simulink model "isi_correlator"  (Simulink 7.0)
settings: Solver=ode45  StartTime=0.0  StopTime=2500  RelTol=1e-3
undefined symbols (not defined in the file): bit_rev, pfb_coeff_gen_calc
libraries: xbsIndex_r4 x4490, casper_library x399, xbsReplacements_r4 x27, isi_correlator_lib x8, xps_library x7, dspsigops x2, simulink x1
(part 2 of 49)

== system isi_correlator/cram2 ==
  In1 : Inport  OutDataType=sfix(16)  OutScaling=2^0
  In2 : Inport  Port=2  OutDataType=sfix(16)  OutScaling=2^0
  In3 : Inport  Port=3  OutDataType=sfix(16)  OutScaling=2^0
  In4 : Inport  Port=4
  In5 : Inport  Port=5
  In6 : Inport  Port=6
  In7 : Inport  Port=7
  In8 : Inport  Port=8
  In9 : Inport  Port=9
  Concat : xbsIndex_r4/Concat  num_inputs=9  dbl_ovrd=off  block_type=concat
      settings: block_version=10.1.2
  Reinterp{1..9} (9 alike) : xbsIndex_r4/Reinterpret  force_arith_type=on  arith_type=Unsigned  force_bin_pt=on  bin_pt=0  block_type=reinterpret
      Reinterp1 settings: infoedit="Changes signal type without altering the binary representation.   You can changed the signal between signed and unsigned, and relocate the binary point.<P><P>Hardware notes: In hardware this block costs nothing.<P><P>Example:  Suppose the input is 6 bits wide, signed, with 2 fractional bits, and the output is forced to unsigned with 0 fractional bits.  Then an input of -2.0 (1110.00 in binary 2's complement) becomes an output of 56 (111000 in binary)."  block_version=10.1.2
      Reinterp2 settings: infoedit="Changes signal type without altering the binary representation.   You can changed the signal between signed and unsigned, and relocate the binary point.<P><P>Hardware notes: In hardware this block costs nothing.<P><P>Example:  Suppose the input is 6 bits wide, signed, with 2 fractional bits, and the output is forced to unsigned with 0 fractional bits.  Then an input of -2.0 (1110.00 in binary 2's complement) becomes an output of 56 (111000 in binary)."  block_version=10.1.2
      Reinterp3 settings: infoedit="Changes signal type without altering the binary representation.   You can changed the signal between signed and unsigned, and relocate the binary point.<P><P>Hardware notes: In hardware this block costs nothing.<P><P>Example:  Suppose the input is 6 bits wide, signed, with 2 fractional bits, and the output is forced to unsigned with 0 fractional bits.  Then an input of -2.0 (1110.00 in binary 2's complement) becomes an output of 56 (111000 in binary)."  block_version=10.1.2
      Reinterp4 settings: infoedit="Changes signal type without altering the binary representation.   You can changed the signal between signed and unsigned, and relocate the binary point.<P><P>Hardware notes: In hardware this block costs nothing.<P><P>Example:  Suppose the input is 6 bits wide, signed, with 2 fractional bits, and the output is forced to unsigned with 0 fractional bits.  Then an input of -2.0 (1110.00 in binary 2's complement) becomes an output of 56 (111000 in binary)."  block_version=10.1.2
      Reinterp5 settings: infoedit="Changes signal type without altering the binary representation.   You can changed the signal between signed and unsigned, and relocate the binary point.<P><P>Hardware notes: In hardware this block costs nothing.<P><P>Example:  Suppose the input is 6 bits wide, signed, with 2 fractional bits, and the output is forced to unsigned with 0 fractional bits.  Then an input of -2.0 (1110.00 in binary 2's complement) becomes an output of 56 (111000 in binary)."  block_version=10.1.3
      Reinterp6 settings: infoedit="Changes signal type without altering the binary representation.   You can changed the signal between signed and unsigned, and relocate the binary point.<P><P>Hardware notes: In hardware this block costs nothing.<P><P>Example:  Suppose the input is 6 bits wide, signed, with 2 fractional bits, and the output is forced to unsigned with 0 fractional bits.  Then an input of -2.0 (1110.00 in binary 2's complement) becomes an output of 56 (111000 in binary)."  block_version=10.1.3
      Reinterp7 settings: infoedit="Changes signal type without altering the binary representation.   You can changed the signal between signed and unsigned, and relocate the binary point.<P><P>Hardware notes: In hardware this block costs nothing.<P><P>Example:  Suppose the input is 6 bits wide, signed, with 2 fractional bits, and the output is forced to unsigned with 0 fractional bits.  Then an input of -2.0 (1110.00 in binary 2's complement) becomes an output of 56 (111000 in binary)."  block_version=10.1.3
      Reinterp8 settings: infoedit="Changes signal type without altering the binary representation.   You can changed the signal between signed and unsigned, and relocate the binary point.<P><P>Hardware notes: In hardware this block costs nothing.<P><P>Example:  Suppose the input is 6 bits wide, signed, with 2 fractional bits, and the output is forced to unsigned with 0 fractional bits.  Then an input of -2.0 (1110.00 in binary 2's complement) becomes an output of 56 (111000 in binary)."  block_version=10.1.3
      Reinterp9 settings: infoedit="Changes signal type without altering the binary representation.   You can changed the signal between signed and unsigned, and relocate the binary point.<P><P>Hardware notes: In hardware this block costs nothing.<P><P>Example:  Suppose the input is 6 bits wide, signed, with 2 fractional bits, and the output is forced to unsigned with 0 fractional bits.  Then an input of -2.0 (1110.00 in binary 2's complement) becomes an output of 56 (111000 in binary)."  block_version=10.1.3
  Out : Outport
  wires:
  Concat/1 -> Out/1
  In1/1 -> Reinterp1/1
  Reinterp1/1 -> Concat/1
  In2/1 -> Reinterp2/1
  Reinterp2/1 -> Concat/2
  In3/1 -> Reinterp3/1
  Reinterp3/1 -> Concat/3
  In4/1 -> Reinterp4/1
  Reinterp4/1 -> Concat/4
  In5/1 -> Reinterp5/1
  Reinterp5/1 -> Concat/5
  In6/1 -> Reinterp6/1
  Reinterp6/1 -> Concat/6
  In7/1 -> Reinterp7/1
  Reinterp7/1 -> Concat/7
  In8/1 -> Reinterp8/1
  Reinterp8/1 -> Concat/8
  In9/1 -> Reinterp9/1
  Reinterp9/1 -> Concat/9

== system isi_correlator/fft_wideband_real ==
  sync : Inport  OutDataType=sfix(16)  OutScaling=2^0
  shift : Inport  Port=2  OutDataType=sfix(16)  OutScaling=2^0
  in0 : Inport  Port=3  OutDataType=sfix(16)  OutScaling=2^0
  in1 : Inport  Port=4  OutDataType=sfix(16)  OutScaling=2^0
  in2 : Inport  Port=5  OutDataType=sfix(16)  OutScaling=2^0
  in3 : Inport  Port=6  OutDataType=sfix(16)  OutScaling=2^0
  in4 : Inport  Port=7
  in5 : Inport  Port=8
  in6 : Inport  Port=9
  in7 : Inport  Port=10
  in8 : Inport  Port=11
  in9 : Inport  Port=12
  in10 : Inport  Port=13
  in11 : Inport  Port=14
  in12 : Inport  Port=15
  in13 : Inport  Port=16
  in14 : Inport  Port=17
  in15 : Inport  Port=18
  fft_biplex_real_4x0 : Subsystem  AncestorBlock=casper_library/FFTs/fft_biplex_real_4x  mask(fft_biplex_real_4x){FFTSize=3, BitWidth=18, quantization=Truncate, overflow=Wrap, add_latency=2, mult_latency=2, bram_latency=3}
      mask prompts: FFTSize "Size of FFT: (2^? pnts)"; BitWidth "Bitwidth: (Max Efficiency at 18 bits)"; quantization "Quantization Behavior"; overflow "Overflow Behavior"; add_latency "Add Latency"; mult_latency "Mult Latency"; bram_latency "BRAM Latency"
      mask init:
      | fft_biplex_real_4x_init(gcb,...
      |     'FFTSize', FFTSize,...
      |     'BitWidth', BitWidth,...
      |     'quantization', quantization,...
      |     'overflow', overflow,...
      |     'add_latency', add_latency,...
      |     'mult_latency', mult_latency,...
      |     'bram_latency', bram_latency);
      settings: AttributesFormatString=FFTSize=3, BitWidth=18
      mask StyleString: edit,edit,popup(Truncate|Round  (unbiased: +/- Inf)|Round  (unbiased: Even Values)),popup(Wrap|Saturate|Error),edit,edit,edit
  fft_biplex_real_4x1 : Subsystem  AncestorBlock=casper_library/FFTs/fft_biplex_real_4x  mask(fft_biplex_real_4x){FFTSize=3, BitWidth=18, quantization=Truncate, overflow=Wrap, add_latency=2, mult_latency=2, bram_latency=3}
      mask prompts: FFTSize "Size of FFT: (2^? pnts)"; BitWidth "Bitwidth: (Max Efficiency at 18 bits)"; quantization "Quantization Behavior"; overflow "Overflow Behavior"; add_latency "Add Latency"; mult_latency "Mult Latency"; bram_latency "BRAM Latency"
      mask init: same as isi_correlator/fft_wideband_real/fft_biplex_real_4x0
      settings: AttributesFormatString=FFTSize=3, BitWidth=18
      mask StyleString: edit,edit,popup(Truncate|Round  (unbiased: +/- Inf)|Round  (unbiased: Even Values)),popup(Wrap|Saturate|Error),edit,edit,edit
  fft_biplex_real_4x2 : Subsystem  AncestorBlock=casper_library/FFTs/fft_biplex_real_4x  mask(fft_biplex_real_4x){FFTSize=3, BitWidth=18, quantization=Truncate, overflow=Wrap, add_latency=2, mult_latency=2, bram_latency=3}
      mask prompts: FFTSize "Size of FFT: (2^? pnts)"; BitWidth "Bitwidth: (Max Efficiency at 18 bits)"; quantization "Quantization Behavior"; overflow "Overflow Behavior"; add_latency "Add Latency"; mult_latency "Mult Latency"; bram_latency "BRAM Latency"
      mask init: same as isi_correlator/fft_wideband_real/fft_biplex_real_4x0
      settings: AttributesFormatString=FFTSize=3, BitWidth=18
      mask StyleString: edit,edit,popup(Truncate|Round  (unbiased: +/- Inf)|Round  (unbiased: Even Values)),popup(Wrap|Saturate|Error),edit,edit,edit
  fft_biplex_real_4x3 : Subsystem  AncestorBlock=casper_library/FFTs/fft_biplex_real_4x  mask(fft_biplex_real_4x){FFTSize=3, BitWidth=18, quantization=Truncate, overflow=Wrap, add_latency=2, mult_latency=2, bram_latency=3}
      mask prompts: FFTSize "Size of FFT: (2^? pnts)"; BitWidth "Bitwidth: (Max Efficiency at 18 bits)"; quantization "Quantization Behavior"; overflow "Overflow Behavior"; add_latency "Add Latency"; mult_latency "Mult Latency"; bram_latency "BRAM Latency"
      mask init: same as isi_correlator/fft_wideband_real/fft_biplex_real_4x0
      settings: AttributesFormatString=FFTSize=3, BitWidth=18
      mask StyleString: edit,edit,popup(Truncate|Round  (unbiased: +/- Inf)|Round  (unbiased: Even Values)),popup(Wrap|Saturate|Error),edit,edit,edit
  fft_direct : Subsystem  AncestorBlock=casper_library/FFTs/fft_direct  mask(fft_direct){FFTSize=4, BitWidth=18, add_latency=2, mult_latency=2, bram_latency=3, quantization=Truncate, overflow=Wrap, MapTail=1, LargerFFTSize=7, StartStage=4}
      mask prompts: FFTSize "Size of FFT: (2^?)"; BitWidth "Bit Width"; add_latency "Add Latency"; mult_latency "Mult Latency"; bram_latency "BRAM Latency"; quantization "Quantization Behavior"; overflow "Overflow Behavior"; MapTail "Map Tail of Larger FFT"; LargerFFTSize "Size of Larger FFT (2^?):"; StartStage "Start Stage of Map Tail:"
      mask init:
      | fft_direct_init(gcb,...
      |     'FFTSize', FFTSize,...
      |     'BitWidth', BitWidth,...
      |     'add_latency', add_latency,...
      |     'mult_latency', mult_latency,...
      |     'bram_latency', bram_latency,...
      |     'quantization', quantization,...
      |     'overflow', overflow,...
      |     'MapTail', MapTail,...
      |     'LargerFFTSize', LargerFFTSize,...
      |     'StartStage', StartStage);
      settings: AttributesFormatString=FFTSize=4
      mask StyleString: edit,edit,edit,edit,edit,popup(Truncate|Round  (unbiased: +/- Inf)|Round  (unbiased: Even Values)),popup(Wrap|Saturate|Error),edit,edit,edit
  fft_unscrambler : Subsystem  AncestorBlock=casper_library/FFTs/fft_unscrambler  mask(fft_unscrambler){FFTSize=6, n_inputs=3, bram_latency=3}
      mask prompts: FFTSize "Size of FFT: (2^?)"; n_inputs "Number of Simultaneous Inputs: (2^?)"; bram_latency "BRAM Latency"
      mask init:
      | fft_unscrambler_init(gcb,...
      |     'FFTSize',FFTSize,... 
      |     'n_inputs', n_inputs,...
      |     'bram_latency', bram_latency);
      settings: AttributesFormatString=FFTSize=6, n_inputs=3
  slice : xbsIndex_r4/Slice  nbits=4  boolean_output=off  mode=Lower Bit Location + Width  bit1=0  base1=MSB of Input  bit0=3  base0=LSB of Input  dbl_ovrd=off  block_type=slice
      settings: infoedit="Extracts a given range of bits from each input sample and presents it at the output.  The output type is ordinarily unsigned with binary point at zero, but can be Boolean when the slice is one bit wide.<P><P>Hardware notes: In hardware this block costs nothing."  block_version=9.1.01
  sync_out : Outport
  out0 : Outport  Port=2
  out1 : Outport  Port=3
  out2 : Outport  Port=4
  out3 : Outport  Port=5
  out4 : Outport  Port=6
  out5 : Outport  Port=7
  out6 : Outport  Port=8
  out7 : Outport  Port=9
  wires:
  in0/1 -> fft_biplex_real_4x0/1(pol1)
  in1/1 -> fft_biplex_real_4x0/2(pol2)
  in2/1 -> fft_biplex_real_4x0/3(pol3)
  in3/1 -> fft_biplex_real_4x0/4(pol4)
  shift/1 -> fft_biplex_real_4x0/6(shift), fft_biplex_real_4x1/6(shift), fft_biplex_real_4x2/6(shift), fft_biplex_real_4x3/6(shift), slice/1
  sync/1 -> fft_biplex_real_4x0/5(sync), fft_biplex_real_4x1/5(sync), fft_biplex_real_4x2/5(sync), fft_biplex_real_4x3/5(sync)
  in4/1 -> fft_biplex_real_4x1/1(pol1)
  in5/1 -> fft_biplex_real_4x1/2(pol2)
  in6/1 -> fft_biplex_real_4x1/3(pol3)
  in7/1 -> fft_biplex_real_4x1/4(pol4)
  in8/1 -> fft_biplex_real_4x2/1(pol1)
  in9/1 -> fft_biplex_real_4x2/2(pol2)
  in10/1 -> fft_biplex_real_4x2/3(pol3)
  in11/1 -> fft_biplex_real_4x2/4(pol4)
  in12/1 -> fft_biplex_real_4x3/1(pol1)
  in13/1 -> fft_biplex_real_4x3/2(pol2)
  in14/1 -> fft_biplex_real_4x3/3(pol3)
  in15/1 -> fft_biplex_real_4x3/4(pol4)
  slice/1 -> fft_direct/2(shift)
  fft_biplex_real_4x0/6(sync_out) -> fft_direct/1(sync)
  fft_biplex_real_4x0/1(pol1_out) -> fft_direct/3(in0)
  fft_biplex_real_4x0/2(pol2_out) -> fft_direct/4(in1)
  fft_biplex_real_4x0/3(pol3_out) -> fft_direct/5(in2)
  fft_biplex_real_4x0/4(pol4_out) -> fft_direct/6(in3)
  fft_biplex_real_4x1/1(pol1_out) -> fft_direct/7(in4)
  fft_biplex_real_4x1/2(pol2_out) -> fft_direct/8(in5)
  fft_biplex_real_4x1/3(pol3_out) -> fft_direct/9(in6)
  fft_biplex_real_4x1/4(pol4_out) -> fft_direct/10(in7)
  fft_biplex_real_4x2/1(pol1_out) -> fft_direct/11(in8)
  fft_biplex_real_4x2/2(pol2_out) -> fft_direct/12(in9)
  fft_biplex_real_4x2/3(pol3_out) -> fft_direct/13(in10)
  fft_biplex_real_4x2/4(pol4_out) -> fft_direct/14(in11)
  fft_biplex_real_4x3/1(pol1_out) -> fft_direct/15(in12)
  fft_biplex_real_4x3/2(pol2_out) -> fft_direct/16(in13)
  fft_biplex_real_4x3/3(pol3_out) -> fft_direct/17(in14)
  fft_biplex_real_4x3/4(pol4_out) -> fft_direct/18(in15)
  fft_direct/1(sync_out) -> fft_unscrambler/1(sync)
  fft_unscrambler/1(sync_out) -> sync_out/1
  fft_direct/2(out0) -> fft_unscrambler/2(In1)
  fft_unscrambler/2(Out1) -> out0/1
  fft_direct/3(out1) -> fft_unscrambler/3(In2)
  fft_unscrambler/3(Out2) -> out1/1
  fft_direct/4(out2) -> fft_unscrambler/4(In3)
  fft_unscrambler/4(Out3) -> out2/1
  fft_direct/5(out3) -> fft_unscrambler/5(In4)
  fft_unscrambler/5(Out4) -> out3/1
  fft_direct/6(out4) -> fft_unscrambler/6(In5)
  fft_unscrambler/6(Out5) -> out4/1
  fft_direct/7(out5) -> fft_unscrambler/7(In6)
  fft_unscrambler/7(Out6) -> out5/1
  fft_direct/8(out6) -> fft_unscrambler/8(In7)
  fft_unscrambler/8(Out7) -> out6/1
  fft_direct/9(out7) -> fft_unscrambler/9(In8)
  fft_unscrambler/9(Out8) -> out7/1

== system isi_correlator/fifo0 ==
  data : Inport
  valid : Inport  Port=2
  Concat : xbsIndex_r4/Concat  num_inputs=2  dbl_ovrd=off  block_type=concat
      settings: block_version=10.1.3
  Constant1 : xbsIndex_r4/Constant  arith_type=Boolean  const=0  n_bits=1  bin_pt=0  explicit_period=on  period=1  equ=P=C  opselect=C  inp2=PCIN>>17  opr=+  inp1=P  carry=CIN  dbl_ovrd=off  block_type=constant
      settings: block_version=10.1.1
  Constant2 : xbsIndex_r4/Constant  arith_type=Unsigned  const=0  n_bits=4  bin_pt=0  explicit_period=on  period=1  equ=P=C  opselect=C  inp2=PCIN>>17  opr=+  inp1=P  carry=CIN  dbl_ovrd=off  block_type=constant
      settings: block_version=10.1.1
  Constant4 : xbsIndex_r4/Constant  arith_type=Boolean  const=0  n_bits=1  bin_pt=0  explicit_period=on  period=1  equ=P=C  opselect=C  inp2=PCIN>>17  opr=+  inp1=P  carry=CIN  dbl_ovrd=off  block_type=constant
      settings: block_version=10.1.1
  Terminator4 : Terminator
  Terminator5 : Terminator
  fifo : xps_library/Shared FIFO  Tag=xps:fifo  SystemSampleTime=-1  io_dir=To Processor  arith_type=Unsigned  data_width=256  data_bin_pt=0  fifo_length=512
      link override convert_isi_correlator_fifo0_fifo_user_data_in: arith_type=Unsigned  n_bits=256  bin_pt=0
      link override convert_isi_correlator_fifo0_fifo_user_level: arith_type=Unsigned  n_bits=16  bin_pt=0
      link override convert_isi_correlator_fifo0_fifo_user_reset: arith_type=Unsigned  n_bits=1  bin_pt=0
      link override convert_isi_correlator_fifo0_fifo_user_we: arith_type=Unsigned  n_bits=1  bin_pt=0
      link override convert_untitled_fifo0_fifo_user_data_in: arith_type=Unsigned  n_bits=256  bin_pt=0
      link override convert_untitled_fifo0_fifo_user_level: arith_type=Unsigned  n_bits=16  bin_pt=0
      link override convert_untitled_fifo0_fifo_user_reset: arith_type=Unsigned  n_bits=1  bin_pt=0
      link override convert_untitled_fifo0_fifo_user_we: arith_type=Unsigned  n_bits=1  bin_pt=0
      link override isi_correlator_fifo0_fifo_user_full: arith_type=Unsigned  n_bits=1  bin_pt=0
      link override isi_correlator_fifo0_fifo_user_level_reached: arith_type=Unsigned  n_bits=1  bin_pt=0
      link override untitled_fifo0_fifo_user_full: arith_type=Unsigned  n_bits=1  bin_pt=0
      link override untitled_fifo0_fifo_user_level_reached: arith_type=Unsigned  n_bits=1  bin_pt=0
  wires:
  Concat/1 -> fifo/1
  valid/1 -> fifo/2
  Constant2/1 -> Concat/2
  data/1 -> Concat/1
  Constant4/1 -> fifo/4
  Constant1/1 -> fifo/3
  fifo/2 -> Terminator4/1
  fifo/1 -> Terminator5/1

== system isi_correlator/fifo1 ==
  data : Inport
  valid : Inport  Port=2
  Concat : xbsIndex_r4/Concat  num_inputs=2  dbl_ovrd=off  block_type=concat
      settings: block_version=10.1.3
  Constant1 : xbsIndex_r4/Constant  arith_type=Boolean  const=0  n_bits=1  bin_pt=0  explicit_period=on  period=1  equ=P=C  opselect=C  inp2=PCIN>>17  opr=+  inp1=P  carry=CIN  dbl_ovrd=off  block_type=constant
      settings: block_version=10.1.1
  Constant2 : xbsIndex_r4/Constant  arith_type=Unsigned  const=0  n_bits=4  bin_pt=0  explicit_period=on  period=1  equ=P=C  opselect=C  inp2=PCIN>>17  opr=+  inp1=P  carry=CIN  dbl_ovrd=off  block_type=constant
      settings: block_version=10.1.1
  Constant4 : xbsIndex_r4/Constant  arith_type=Boolean  const=0  n_bits=1  bin_pt=0  explicit_period=on  period=1  equ=P=C  opselect=C  inp2=PCIN>>17  opr=+  inp1=P  carry=CIN  dbl_ovrd=off  block_type=constant
      settings: block_version=10.1.1
  Terminator4 : Terminator
  Terminator5 : Terminator
  fifo1 : xps_library/Shared FIFO  Tag=xps:fifo  SystemSampleTime=-1  io_dir=To Processor  arith_type=Unsigned  data_width=256  data_bin_pt=0  fifo_length=512
      link override convert_isi_correlator_fifo1_fifo1_user_data_in: arith_type=Unsigned  n_bits=256  bin_pt=0
      link override convert_isi_correlator_fifo1_fifo1_user_level: arith_type=Unsigned  n_bits=16  bin_pt=0
      link override convert_isi_correlator_fifo1_fifo1_user_reset: arith_type=Unsigned  n_bits=1  bin_pt=0
      link override convert_isi_correlator_fifo1_fifo1_user_we: arith_type=Unsigned  n_bits=1  bin_pt=0
      link override convert_untitled_fifo1_fifo1_user_data_in: arith_type=Unsigned  n_bits=256  bin_pt=0
      link override convert_untitled_fifo1_fifo1_user_level: arith_type=Unsigned  n_bits=16  bin_pt=0
      link override convert_untitled_fifo1_fifo1_user_reset: arith_type=Unsigned  n_bits=1  bin_pt=0
      link override convert_untitled_fifo1_fifo1_user_we: arith_type=Unsigned  n_bits=1  bin_pt=0
      link override isi_correlator_fifo1_fifo1_user_full: arith_type=Unsigned  n_bits=1  bin_pt=0
      link override isi_correlator_fifo1_fifo1_user_level_reached: arith_type=Unsigned  n_bits=1  bin_pt=0
      link override untitled_fifo1_fifo1_user_full: arith_type=Unsigned  n_bits=1  bin_pt=0
      link override untitled_fifo1_fifo1_user_level_reached: arith_type=Unsigned  n_bits=1  bin_pt=0
  wires:
  Concat/1 -> fifo1/1
  valid/1 -> fifo1/2
  Constant2/1 -> Concat/2
  data/1 -> Concat/1
  Constant4/1 -> fifo1/4
  Constant1/1 -> fifo1/3
  fifo1/2 -> Terminator4/1
  fifo1/1 -> Terminator5/1

== system isi_correlator/fifo2 ==
  data : Inport
  valid : Inport  Port=2
  Concat : xbsIndex_r4/Concat  num_inputs=2  dbl_ovrd=off  block_type=concat
      settings: block_version=10.1.3
  Constant1 : xbsIndex_r4/Constant  arith_type=Boolean  const=0  n_bits=1  bin_pt=0  explicit_period=on  period=1  equ=P=C  opselect=C  inp2=PCIN>>17  opr=+  inp1=P  carry=CIN  dbl_ovrd=off  block_type=constant
      settings: block_version=10.1.1
  Constant2 : xbsIndex_r4/Constant  arith_type=Unsigned  const=0  n_bits=4  bin_pt=0  explicit_period=on  period=1  equ=P=C  opselect=C  inp2=PCIN>>17  opr=+  inp1=P  carry=CIN  dbl_ovrd=off  block_type=constant
      settings: block_version=10.1.1
  Constant4 : xbsIndex_r4/Constant  arith_type=Boolean  const=0  n_bits=1  bin_pt=0  explicit_period=on  period=1  equ=P=C  opselect=C  inp2=PCIN>>17  opr=+  inp1=P  carry=CIN  dbl_ovrd=off  block_type=constant
      settings: block_version=10.1.1
  Terminator4 : Terminator
  Terminator5 : Terminator
  fifo2 : xps_library/Shared FIFO  Tag=xps:fifo  SystemSampleTime=-1  io_dir=To Processor  arith_type=Unsigned  data_width=256  data_bin_pt=0  fifo_length=512
      link override convert_isi_correlator_fifo2_fifo2_user_data_in: arith_type=Unsigned  n_bits=256  bin_pt=0
      link override convert_isi_correlator_fifo2_fifo2_user_level: arith_type=Unsigned  n_bits=16  bin_pt=0
      link override convert_isi_correlator_fifo2_fifo2_user_reset: arith_type=Unsigned  n_bits=1  bin_pt=0
      link override convert_isi_correlator_fifo2_fifo2_user_we: arith_type=Unsigned  n_bits=1  bin_pt=0
      link override convert_untitled_fifo2_fifo2_user_data_in: arith_type=Unsigned  n_bits=256  bin_pt=0
      link override convert_untitled_fifo2_fifo2_user_level: arith_type=Unsigned  n_bits=16  bin_pt=0
      link override convert_untitled_fifo2_fifo2_user_reset: arith_type=Unsigned  n_bits=1  bin_pt=0
      link override convert_untitled_fifo2_fifo2_user_we: arith_type=Unsigned  n_bits=1  bin_pt=0
      link override isi_correlator_fifo2_fifo2_user_full: arith_type=Unsigned  n_bits=1  bin_pt=0
      link override isi_correlator_fifo2_fifo2_user_level_reached: arith_type=Unsigned  n_bits=1  bin_pt=0
      link override untitled_fifo2_fifo2_user_full: arith_type=Unsigned  n_bits=1  bin_pt=0
      link override untitled_fifo2_fifo2_user_level_reached: arith_type=Unsigned  n_bits=1  bin_pt=0
  wires:
  Concat/1 -> fifo2/1
  valid/1 -> fifo2/2
  Constant2/1 -> Concat/2
  data/1 -> Concat/1
  Constant4/1 -> fifo2/4
  Constant1/1 -> fifo2/3
  fifo2/2 -> Terminator4/1
  fifo2/1 -> Terminator5/1
